simulink model "Simulatore_COMAU_UBHAND_IV_manipolazione_interna"  (Simulink 7.6)
settings: Solver=ode45  StartTime=0.0  StopTime=inf  RelTol=1e-3
values from neighbouring files (symbols this model uses):
  a_f = zeros(4,5)    [KinematicData.m]
  alpha_f = zeros(4,5)    [KinematicData.m]
  d_f = zeros(4,5)    [KinematicData.m]
  dc4_1 = 660    [KinematicData.m]
  T6HAND = [1 0 0 0;0 1 0 0; 0 0 1 357.48+95; 0 0 0 1]    [KinematicData.m]
  teta_d = atan(647.07/130)    [KinematicData.m]
  teta_f = zeros(4,5)    [KinematicData.m]
undefined symbols (not defined in the file or in its folder): a_c, a_w, alpha_c, alpha_w, d_c, d_w, Dt_fingers, teta_c
libraries: simulink x281, dspmtrx3 x171, aerolibutil x163, aerolibconvert2 x64, simulink_extras x46, vrlib x26, aerolibtransform2 x7, dspnetwork x1
(part 4 of 15)

== system Simulatore_COMAU_UBHAND_IV_manipolazione_interna/SCOPES/Direct_kinematic2/Direct_kinematic_hand/Direct_kinematic_thumb ==
  Joints_reference : Inport  PortDimensions=3
  T_0f : Inport  Port=2
  Add{,1..4} (5 alike) : Sum
  Constant : Constant  Value=[alpha_f(1,1) alpha_f(1,2) alpha_f(1,3) alpha_f(1,4)]
  Constant1 : Constant  Value=teta_f(1,1)
  Constant2 : Constant  Value=[a_f(1,1) a_f(1,2) a_f(1,3) a_f(1,4)]
  Constant3 : Constant  Value=teta_f(1,2)
  Constant4 : Constant  Value=teta_f(1,3)
  Constant5 : Constant  Value=teta_f(1,4)
  Constant6 : Constant  Value=-20
  Degrees to Radians : simulink_extras/Transformations/Degrees to Radians
  Demux : Demux
  Demux1 : Demux
  Demux2 : Demux
  Demux3 : Demux  Outputs=3
  Display3 : Display
  Gain : Gain  Gain=0.8
  Matrix Multiply{,1..3} (4 alike) : Product  Multiplication=Matrix(*)
  Subsystem : Subsystem
  Subsystem1 : Subsystem
  Subsystem2 : Subsystem
  Subsystem3 : Subsystem
  Vector Concatenate : Concatenate  Mode=Vector
  T_finger_tip : Outport
  T_thumb_base : Outport  Port=2
  wires:
  Constant/1 -> Demux1/1
  Subsystem/1(Out1) -> Matrix Multiply3/2
  Subsystem1/1(Out1) -> Matrix Multiply/2
  Matrix Multiply/1 -> Matrix Multiply1/1
  Subsystem2/1(Out1) -> Matrix Multiply1/2
  Subsystem3/1(Out1) -> Matrix Multiply2/2
  Matrix Multiply1/1 -> Matrix Multiply2/1
  Matrix Multiply2/1 -> T_finger_tip/1, T_thumb_base/1
  Constant2/1 -> Demux2/1
  Demux2/1 -> Subsystem/3(a)
  Demux2/2 -> Subsystem1/3(a)
  Demux2/3 -> Subsystem2/3(a)
  Demux2/4 -> Subsystem3/3(a)
  Demux1/1 -> Subsystem/2(alpha)
  Demux1/2 -> Subsystem1/2(alpha)
  Demux1/3 -> Subsystem2/2(alpha)
  Demux1/4 -> Subsystem3/2(alpha)
  Joints_reference/1 -> Demux3/1, Vector Concatenate/1
  Demux3/3 -> Gain/1
  Vector Concatenate/1 -> Degrees to Radians/1, Display3/1
  Degrees to Radians/1 -> Demux/1
  T_0f/1 -> Matrix Multiply3/1
  Matrix Multiply3/1 -> Matrix Multiply/1
  Constant1/1 -> Add/2
  Demux/1 -> Add/1
  Demux/2 -> Add1/1
  Demux/3 -> Add2/1
  Demux/4 -> Add3/1
  Add3/1 -> Subsystem3/1(q)
  Add2/1 -> Subsystem2/1(q)
  Add1/1 -> Subsystem1/1(q)
  Add/1 -> Subsystem/1(q)
  Constant3/1 -> Add1/2
  Constant4/1 -> Add2/2
  Constant5/1 -> Add3/2
  Constant6/1 -> Add4/2
  Gain/1 -> Add4/1
  Add4/1 -> Vector Concatenate/2

== system Simulatore_COMAU_UBHAND_IV_manipolazione_interna/SCOPES/Direct_kinematic2/Direct_kinematic_wrist/T_0_8 ==
  wrist_angles[1..2] : Inport
  T_0HAND : Inport  Port=2
  Add : Sum
  Add1 : Sum
  Constant : Constant  Value=[alpha_w(1) alpha_w(2)]
  Constant1 : Constant  Value=0
  Constant2 : Constant  Value=[a_w(1) a_w(2)]
  Constant3 : Constant  Value=0
  Degrees to Radians : simulink_extras/Transformations/Degrees to Radians
  Demux : Demux  Outputs=2
  Demux1 : Demux  Outputs=2
  Demux2 : Demux  Outputs=2
  Display3 : Display
  Matrix Multiply : Product  Multiplication=Matrix(*)
  Matrix Multiply1 : Product  Multiplication=Matrix(*)
  Matrix Multiply2 : Product  Multiplication=Matrix(*)
  Subsystem : Subsystem
  Subsystem1 : Subsystem
  wrist_reference_ : Subsystem
  T_08 : Outport
  wires:
  wrist_angles[1..2]/1 -> Display3/1, Degrees to Radians/1
  Degrees to Radians/1 -> Demux/1
  Demux1/2 -> Subsystem1/2(alpha)
  Demux1/1 -> Subsystem/2(alpha)
  Demux2/2 -> Subsystem1/3(a)
  Demux2/1 -> Subsystem/3(a)
  Constant2/1 -> Demux2/1
  Matrix Multiply/1 -> T_08/1
  Subsystem1/1(Out1) -> Matrix Multiply/2
  Constant/1 -> Demux1/1
  Subsystem/1(Out1) -> Matrix Multiply1/2
  Matrix Multiply1/1 -> Matrix Multiply/1
  Constant1/1 -> Add/2
  Demux/1 -> Add/1
  Add/1 -> Subsystem/1(q)
  Constant3/1 -> Add1/2
  Demux/2 -> Add1/1
  Add1/1 -> Subsystem1/1(q)
  T_0HAND/1 -> Matrix Multiply2/1
  wrist_reference_/1(T0_W) -> Matrix Multiply2/2
  Matrix Multiply2/1 -> Matrix Multiply1/1

== system Simulatore_COMAU_UBHAND_IV_manipolazione_interna/VISUALIZATION/Subsystem3/Subsystem1/Wrist ==
  xyz_0 : Inport
  Constant : Constant  Value=0
  Constant1 : Constant  Value=0
  Constant2 : Constant  Value=0
  Demux : Demux  Outputs=3
  Display : Display
  Display1 : Display
  Gain : Gain  Gain=-1
  Gain1 : Gain  Gain=-1
  Gain2 : Gain
  Mux : Mux  Inputs=3
  Sum : Sum
  Sum1 : Sum
  Sum2 : Sum
  xyz_vrl : Outport
  wires:
  Gain1/1 -> Mux/1
  Gain2/1 -> Mux/2
  Gain/1 -> Mux/3
  Sum2/1 -> Gain2/1
  Sum1/1 -> Gain1/1
  Sum/1 -> Gain/1
  Mux/1 -> Display/1, xyz_vrl/1
  Constant1/1 -> Sum1/1
  Demux/2 -> Sum1/2
  Constant2/1 -> Sum2/1
  Demux/3 -> Sum2/2
  Constant/1 -> Sum/1
  Demux/1 -> Sum/2
  xyz_0/1 -> Demux/1, Display1/1

== system Simulatore_COMAU_UBHAND_IV_manipolazione_interna/VISUALIZATION/Subsystem3/Subsystem2/Subsystem ==
  carpale_in : Inport
  prossimale_in : Inport  Port=2
  mediale_in : Inport  Port=3
  Angle Conversion : aerolibconvert2/Angle Conversion  IU=deg  OU=rad
  Angle Conversion1 : aerolibconvert2/Angle Conversion  IU=deg  OU=rad
  Constant : Constant
  Constant1 : Constant  Value=-1
  Constant{2..5} (4 alike) : Constant  Value=0
  Constant6 : Constant  Value=-90
  Constant7 : Constant  Value=20
  Gain : Gain  Gain=0.8
  Mux{1..4} (4 alike) : Mux
  Sum : Sum  Inputs=|++
  Sum1 : Sum  Inputs=|+-
  carpale_out : Outport
  prossimale_out : Outport  Port=2
  mediale_out : Outport  Port=3
  distale_out : Outport  Port=4
  wires:
  Constant/1 -> [uno]
  Constant1/1 -> [meno_uno]
  mediale_in/1 -> Mux3/4, Gain/1
  [uno] -> Mux3/2
  Constant4/1 -> Mux3/1, Mux3/3
  Mux3/1 -> mediale_out/1
  [uno] -> Mux4/2
  Constant5/1 -> Mux4/1, Mux4/3
  Mux4/1 -> distale_out/1
  [uno] -> Mux1/1
  Constant2/1 -> Mux1/3, Mux1/2
  Sum/1 -> Mux1/4
  Constant6/1 -> Angle Conversion/1
  prossimale_in/1 -> Sum/1
  Mux1/1 -> prossimale_out/1
  [meno_uno] -> Mux2/3
  carpale_in/1 -> Mux2/4
  Constant3/1 -> Mux2/1, Mux2/2
  Mux2/1 -> carpale_out/1
  Constant7/1 -> Angle Conversion1/1
  Gain/1 -> Sum1/1
  Sum1/1 -> Mux4/4
  Angle Conversion/1 -> Sum/2
  Angle Conversion1/1 -> Sum1/2

== system Simulatore_COMAU_UBHAND_IV_manipolazione_interna/VISUALIZATION/Subsystem3/Subsystem2/Subsystem1 ==
  carpale_in : Inport
  prossimale_in : Inport  Port=2
  mediale_in : Inport  Port=3
  Constant : Constant
  Constant1 : Constant  Value=-1
  Constant{2..5} (4 alike) : Constant  Value=0
  Display : Display
  Gain : Gain  Gain=0.7
  Mux{1..4} (4 alike) : Mux
  carpale_out : Outport
  prossimale_out : Outport  Port=2
  mediale_out : Outport  Port=3
  distale_out : Outport  Port=4
  wires:
  Mux1/1 -> carpale_out/1
  Mux2/1 -> prossimale_out/1
  Constant/1 -> [uno]
  Constant1/1 -> [meno_uno]
  Constant2/1 -> Mux1/1, Mux1/3
  carpale_in/1 -> Mux1/4
  prossimale_in/1 -> Mux2/4
  mediale_in/1 -> Mux3/4, Gain/1
  Constant4/1 -> Mux3/2, Mux3/3
  Mux3/1 -> mediale_out/1
  Constant5/1 -> Mux4/3, Mux4/2
  Gain/1 -> Mux4/4
  Mux4/1 -> distale_out/1, Display/1
  [meno_uno] -> Mux1/2
  [uno] -> Mux2/1
  Constant3/1 -> Mux2/2, Mux2/3
  [uno] -> Mux3/1
  [uno] -> Mux4/1

== system Simulatore_COMAU_UBHAND_IV_manipolazione_interna/VISUALIZATION/Subsystem3/Subsystem2/Subsystem2 ==
  carpale_in : Inport
  prossimale_in : Inport  Port=2
  mediale_in : Inport  Port=3
  Constant : Constant
  Constant1 : Constant  Value=-1
  Constant{2..5} (4 alike) : Constant  Value=0
  Gain : Gain  Gain=0.7
  Mux{1..4} (4 alike) : Mux
  carpale_out : Outport
  prossimale_out : Outport  Port=2
  mediale_out : Outport  Port=3
  distale_out : Outport  Port=4
  wires:
  Constant/1 -> [uno]
  Constant1/1 -> [meno_uno]
  Mux1/1 -> carpale_out/1
  Mux2/1 -> prossimale_out/1
  Constant2/1 -> Mux1/1, Mux1/3
  carpale_in/1 -> Mux1/4
  prossimale_in/1 -> Mux2/4
  mediale_in/1 -> Mux3/4, Gain/1
  Constant4/1 -> Mux3/2, Mux3/3
  Mux3/1 -> mediale_out/1
  Constant5/1 -> Mux4/3, Mux4/2
  Gain/1 -> Mux4/4
  Mux4/1 -> distale_out/1
  [meno_uno] -> Mux1/2
  [uno] -> Mux2/1
  Constant3/1 -> Mux2/2, Mux2/3
  [uno] -> Mux3/1
  [uno] -> Mux4/1

== system Simulatore_COMAU_UBHAND_IV_manipolazione_interna/VISUALIZATION/Subsystem3/Subsystem2/Subsystem3 ==
  carpale_in : Inport
  prossimale_in : Inport  Port=2
  mediale_in : Inport  Port=3
  Constant : Constant
  Constant1 : Constant  Value=-1
  Constant{2..5} (4 alike) : Constant  Value=0
  Gain : Gain  Gain=0.7
  Mux{1..4} (4 alike) : Mux
  carpale_out : Outport
  prossimale_out : Outport  Port=2
  mediale_out : Outport  Port=3
  distale_out : Outport  Port=4
  wires:
  Constant/1 -> [uno]
  Constant1/1 -> [meno_uno]
  Mux1/1 -> carpale_out/1
  Mux2/1 -> prossimale_out/1
  Constant2/1 -> Mux1/1, Mux1/3
  carpale_in/1 -> Mux1/4
  prossimale_in/1 -> Mux2/4
  mediale_in/1 -> Mux3/4, Gain/1
  Constant4/1 -> Mux3/2, Mux3/3
  Mux3/1 -> mediale_out/1
  Constant5/1 -> Mux4/3, Mux4/2
  Gain/1 -> Mux4/4
  Mux4/1 -> distale_out/1
  [meno_uno] -> Mux1/2
  [uno] -> Mux2/1
  Constant3/1 -> Mux2/2, Mux2/3
  [uno] -> Mux3/1
  [uno] -> Mux4/1

== system Simulatore_COMAU_UBHAND_IV_manipolazione_interna/VISUALIZATION/Subsystem3/Subsystem2/Subsystem4 ==
  carpale_in : Inport
  prossimale_in : Inport  Port=2
  mediale_in : Inport  Port=3
  Constant : Constant
  Constant1 : Constant  Value=-1
  Constant{2..5} (4 alike) : Constant  Value=0
  Gain : Gain  Gain=0.7
  Mux{1..4} (4 alike) : Mux
  carpale_out : Outport
  prossimale_out : Outport  Port=2
  mediale_out : Outport  Port=3
  distale_out : Outport  Port=4
  wires:
  Constant/1 -> [uno]
  Constant1/1 -> [meno_uno]
  Mux1/1 -> carpale_out/1
  Mux2/1 -> prossimale_out/1
  Constant2/1 -> Mux1/1, Mux1/3
  carpale_in/1 -> Mux1/4
  prossimale_in/1 -> Mux2/4
  mediale_in/1 -> Mux3/4, Gain/1
  Constant4/1 -> Mux3/2, Mux3/3
  Mux3/1 -> mediale_out/1
  Constant5/1 -> Mux4/3, Mux4/2
  Gain/1 -> Mux4/4
  Mux4/1 -> distale_out/1
  [meno_uno] -> Mux1/2
  [uno] -> Mux2/1
  Constant3/1 -> Mux2/2, Mux2/3
  [uno] -> Mux3/1
  [uno] -> Mux4/1

== system Simulatore_COMAU_UBHAND_IV_manipolazione_interna/VISUALIZATION/Subsystem3/Subsystem2/Subsystem5 ==
  wrist 1_in : Inport
  wrist 2_in : Inport  Port=2
  Constant : Constant
  Constant1 : Constant  Value=-1
  Constant2 : Constant  Value=0
  Constant3 : Constant  Value=0
  Mux1 : Mux
  Mux2 : Mux
  wrist 1_out : Outport
  wrist 2_out : Outport  Port=2
  wires:
  Constant3/1 -> Mux2/3, Mux2/2
  wrist 2_in/1 -> Mux2/4
  wrist 1_in/1 -> Mux1/4
  Constant2/1 -> Mux1/3, Mux1/2
  Constant1/1 -> [meno_uno]
  Constant/1 -> [uno]
  Mux2/1 -> wrist 2_out/1
  Mux1/1 -> wrist 1_out/1
  [uno] -> Mux1/1
  [meno_uno] -> Mux2/1

== system Simulatore_COMAU_UBHAND_IV_manipolazione_interna/VISUALIZATION/Subsystem3/finger_tip_xyz/world_frame2vrl_frame ==
  xyz_0 : Inport
  Constant : Constant  Value=0
  Constant1 : Constant  Value=0
  Constant2 : Constant  Value=0
  Demux : Demux  Outputs=3
  Display : Display
  Display1 : Display
  Gain : Gain  Gain=-1
  Gain1 : Gain  Gain=-1
  Gain2 : Gain
  Mux : Mux  Inputs=3
  Sum : Sum
  Sum1 : Sum
  Sum2 : Sum
  xyz_vrl : Outport
  wires:
  Gain1/1 -> Mux/1
  Gain2/1 -> Mux/2
  Gain/1 -> Mux/3
  Sum2/1 -> Gain2/1
  Sum1/1 -> Gain1/1
  Sum/1 -> Gain/1
  Mux/1 -> Display/1, xyz_vrl/1
  Constant1/1 -> Sum1/1
  Demux/2 -> Sum1/2
  Constant2/1 -> Sum2/1
  Demux/3 -> Sum2/2
  Constant/1 -> Sum/1
  Demux/1 -> Sum/2
  xyz_0/1 -> Demux/1, Display1/1

== system Simulatore_COMAU_UBHAND_IV_manipolazione_interna/VISUALIZATION/Subsystem3/finger_tip_xyz/world_frame2vrl_frame1 ==
  xyz_0 : Inport
  Constant : Constant  Value=0
  Constant1 : Constant  Value=0
  Constant2 : Constant  Value=0
  Demux : Demux  Outputs=3
  Display : Display
  Display1 : Display
  Gain : Gain  Gain=-1
  Gain1 : Gain  Gain=-1
  Gain2 : Gain
  Mux : Mux  Inputs=3
  Sum : Sum
  Sum1 : Sum
  Sum2 : Sum
  xyz_vrl : Outport
  wires:
  xyz_0/1 -> Display1/1, Demux/1
  Demux/1 -> Sum/2
  Constant/1 -> Sum/1
  Demux/3 -> Sum2/2
  Constant2/1 -> Sum2/1
  Demux/2 -> Sum1/2
  Constant1/1 -> Sum1/1
  Mux/1 -> xyz_vrl/1, Display/1
  Sum/1 -> Gain/1
  Sum1/1 -> Gain1/1
  Sum2/1 -> Gain2/1
  Gain/1 -> Mux/3
  Gain2/1 -> Mux/2
  Gain1/1 -> Mux/1

== system Simulatore_COMAU_UBHAND_IV_manipolazione_interna/VISUALIZATION/Subsystem3/finger_tip_xyz/world_frame2vrl_frame2 ==
  xyz_0 : Inport
  Constant : Constant  Value=0
  Constant1 : Constant  Value=0
  Constant2 : Constant  Value=0
  Demux : Demux  Outputs=3
  Display : Display
  Display1 : Display
  Gain : Gain  Gain=-1
  Gain1 : Gain  Gain=-1
  Gain2 : Gain
  Mux : Mux  Inputs=3
  Sum : Sum
  Sum1 : Sum
  Sum2 : Sum
  xyz_vrl : Outport
  wires:
  Gain1/1 -> Mux/1
  Gain2/1 -> Mux/2
  Gain/1 -> Mux/3
  Sum2/1 -> Gain2/1
  Sum1/1 -> Gain1/1
  Sum/1 -> Gain/1
  Mux/1 -> Display/1, xyz_vrl/1
  Constant1/1 -> Sum1/1
  Demux/2 -> Sum1/2
  Constant2/1 -> Sum2/1
  Demux/3 -> Sum2/2
  Constant/1 -> Sum/1
  Demux/1 -> Sum/2
  xyz_0/1 -> Demux/1, Display1/1

== system Simulatore_COMAU_UBHAND_IV_manipolazione_interna/VISUALIZATION/Subsystem3/finger_tip_xyz/world_frame2vrl_frame3 ==
  xyz_0 : Inport
  Constant : Constant  Value=0
  Constant1 : Constant  Value=0
  Constant2 : Constant  Value=0
  Demux : Demux  Outputs=3
  Display : Display
  Display1 : Display
  Gain : Gain  Gain=-1
  Gain1 : Gain  Gain=-1
  Gain2 : Gain
  Mux : Mux  Inputs=3
  Sum : Sum
  Sum1 : Sum
  Sum2 : Sum
  xyz_vrl : Outport
  wires:
  xyz_0/1 -> Display1/1, Demux/1
  Demux/1 -> Sum/2
  Constant/1 -> Sum/1
  Demux/3 -> Sum2/2
  Constant2/1 -> Sum2/1
  Demux/2 -> Sum1/2
  Constant1/1 -> Sum1/1
  Mux/1 -> xyz_vrl/1, Display/1
  Sum/1 -> Gain/1
  Sum1/1 -> Gain1/1
  Sum2/1 -> Gain2/1
  Gain/1 -> Mux/3
  Gain2/1 -> Mux/2
  Gain1/1 -> Mux/1

== system Simulatore_COMAU_UBHAND_IV_manipolazione_interna/VISUALIZATION/Subsystem3/finger_tip_xyz/world_frame2vrl_frame4 ==
  xyz_0 : Inport
  Constant : Constant  Value=0
  Constant1 : Constant  Value=0
  Constant2 : Constant  Value=0
  Demux : Demux  Outputs=3
  Display : Display
  Display1 : Display
  Gain : Gain  Gain=-1
  Gain1 : Gain  Gain=-1
  Gain2 : Gain
  Mux : Mux  Inputs=3
  Sum : Sum
  Sum1 : Sum
  Sum2 : Sum
  xyz_vrl : Outport
  wires:
  Gain1/1 -> Mux/1
  Gain2/1 -> Mux/2
  Gain/1 -> Mux/3
  Sum2/1 -> Gain2/1
  Sum1/1 -> Gain1/1
  Sum/1 -> Gain/1
  Mux/1 -> Display/1, xyz_vrl/1
  Constant1/1 -> Sum1/1
  Demux/2 -> Sum1/2
  Constant2/1 -> Sum2/1
  Demux/3 -> Sum2/2
  Constant/1 -> Sum/1
  Demux/1 -> Sum/2
  xyz_0/1 -> Demux/1, Display1/1

== system Simulatore_COMAU_UBHAND_IV_manipolazione_interna/Work Space Control/Grasp Planner/SCOPES/Direct_kinematic2 ==
  q[1..6] : Inport
  wrist_angles[1..2] : Inport  Port=2
  fingers_joints_references : Inport  Port=3
  Constant2 : Constant  Value=T6HAND  VectorParams1D=off
  Direct_kinematic_Comau : Subsystem
  Direct_kinematic_hand : Subsystem
  Direct_kinematic_wrist : Subsystem
  Matrix Multiply1 : Product  Multiplication=Matrix(*)
  Mux : Mux  Inputs=5
  T0HAND : Outport
  fingers_tips_xyz : Outport  Port=2
  wires:
  q[1..6]/1 -> Direct_kinematic_Comau/1(q[1..6])
  Direct_kinematic_Comau/1(T_0_6) -> Matrix Multiply1/1
  Constant2/1 -> Matrix Multiply1/2
  Matrix Multiply1/1 -> Direct_kinematic_wrist/2(T_0HAND)
  wrist_angles[1..2]/1 -> Direct_kinematic_wrist/1(wrist_angles[1..2])
  fingers_joints_references/1 -> Direct_kinematic_hand/1(Joint_references)
  Direct_kinematic_wrist/1(T_08) -> Direct_kinematic_hand/2(T_08), T0HAND/1
  Direct_kinematic_hand/1(T_finger_tip_thumb) -> Mux/1
  Direct_kinematic_hand/2(T_finger_tip_index) -> Mux/2
  Direct_kinematic_hand/3(T_finger_tip_middle) -> Mux/3
  Direct_kinematic_hand/4(T_finger_tip_ring) -> Mux/4
  Direct_kinematic_hand/5(T_finger_tip_little) -> Mux/5
  Mux/1 -> fingers_tips_xyz/1

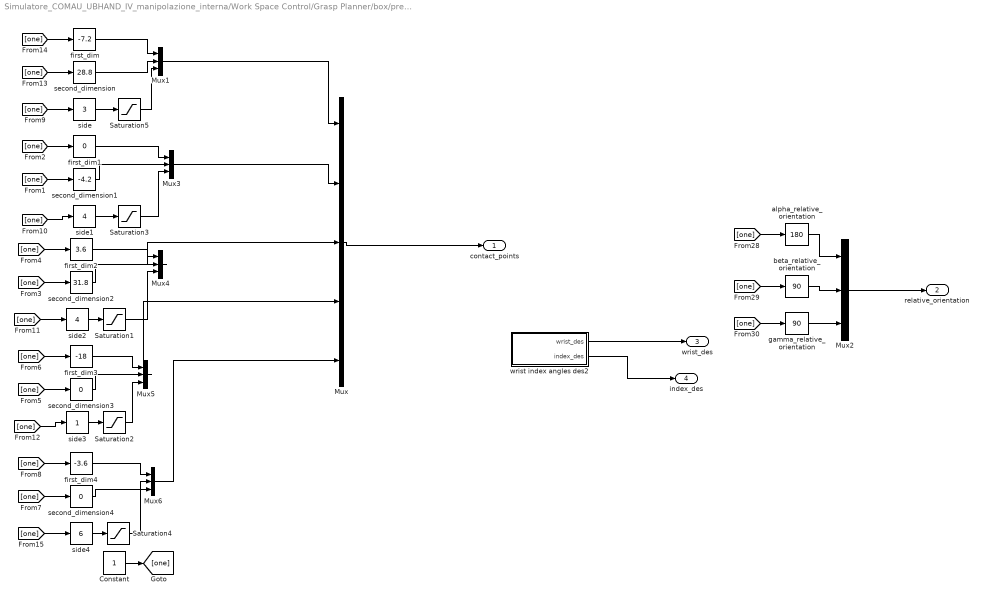
== system Simulatore_COMAU_UBHAND_IV_manipolazione_interna/Work Space Control/Grasp Planner/box/presa da destra ==
  Constant : Constant
  Mux : Mux  Inputs=5
  Mux{1..6} (6 alike) : Mux  Inputs=3
  Saturation{1..5} (5 alike) : Saturate  UpperLimit=6  LowerLimit=1
  alpha_relative_orientation : simulink/Math Operations/Slider Gain  low=-180  gain=180  high=180
  beta_relative_orientation : simulink/Math Operations/Slider Gain  low=-180  gain=90  high=180
  first_dim : simulink/Math Operations/Slider Gain  low=-180  gain=-7.2  high=180
  first_dim1 : simulink/Math Operations/Slider Gain  low=-180  gain=0  high=180
  first_dim2 : simulink/Math Operations/Slider Gain  low=-180  gain=3.6  high=180
  first_dim3 : simulink/Math Operations/Slider Gain  low=-180  gain=-18  high=180
  first_dim4 : simulink/Math Operations/Slider Gain  low=-180  gain=-3.6  high=180
  gamma_relative_orientation : simulink/Math Operations/Slider Gain  low=-180  gain=90  high=180
  second_dimension : simulink/Math Operations/Slider Gain  low=-180  gain=28.8  high=180
  second_dimension1 : simulink/Math Operations/Slider Gain  low=-180  gain=-4.2  high=180
  second_dimension2 : simulink/Math Operations/Slider Gain  low=-180  gain=31.8  high=180
  second_dimension3 : simulink/Math Operations/Slider Gain  low=-180  gain=0  high=180
  second_dimension4 : simulink/Math Operations/Slider Gain  low=-180  gain=0  high=180
  side : simulink/Math Operations/Slider Gain  low=-94  gain=3  high=6
  side1 : simulink/Math Operations/Slider Gain  low=-94  gain=4  high=6
  side2 : simulink/Math Operations/Slider Gain  low=-94  gain=4  high=6
  side3 : simulink/Math Operations/Slider Gain  low=-94  gain=1  high=6
  side4 : simulink/Math Operations/Slider Gain  low=-94  gain=6  high=6
  wrist index angles des2 : Subsystem
  contact_points : Outport
  relative_orientation : Outport  Port=2
  wrist_des : Outport  Port=3
  index_des : Outport  Port=4
  wires:
  Mux2/1 -> relative_orientation/1
  gamma_relative_orientation/1 -> Mux2/3
  beta_relative_orientation/1 -> Mux2/2
  alpha_relative_orientation/1 -> Mux2/1
  [one] -> gamma_relative_orientation/1
  [one] -> beta_relative_orientation/1
  [one] -> alpha_relative_orientation/1
  Constant/1 -> [one]
  wrist index angles des2/1(wrist_des) -> wrist_des/1
  wrist index angles des2/2(index_des) -> index_des/1
  Mux/1 -> contact_points/1
  [one] -> second_dimension/1
  [one] -> first_dim/1
  first_dim/1 -> Mux1/1
  second_dimension/1 -> Mux1/2
  Mux1/1 -> Mux/1
  side/1 -> Saturation5/1
  [one] -> second_dimension1/1
  [one] -> first_dim1/1
  first_dim1/1 -> Mux3/1
  second_dimension1/1 -> Mux3/2
  side1/1 -> Saturation3/1
  [one] -> second_dimension2/1
  [one] -> first_dim2/1
  first_dim2/1 -> Mux4/1
  second_dimension2/1 -> Mux4/2
  side2/1 -> Saturation1/1
  [one] -> second_dimension3/1
  [one] -> first_dim3/1
  first_dim3/1 -> Mux5/1
  second_dimension3/1 -> Mux5/2
  side3/1 -> Saturation2/1
  [one] -> second_dimension4/1
  [one] -> first_dim4/1
  first_dim4/1 -> Mux6/1
  second_dimension4/1 -> Mux6/2
  side4/1 -> Saturation4/1
  Mux3/1 -> Mux/2
  Mux4/1 -> Mux/3
  Mux5/1 -> Mux/4
  Mux6/1 -> Mux/5
  [one] -> side/1
  [one] -> side1/1
  [one] -> side2/1
  [one] -> side3/1
  [one] -> side4/1
  Saturation4/1 -> Mux6/3
  Saturation2/1 -> Mux5/3
  Saturation1/1 -> Mux4/3
  Saturation3/1 -> Mux3/3
  Saturation5/1 -> Mux1/3

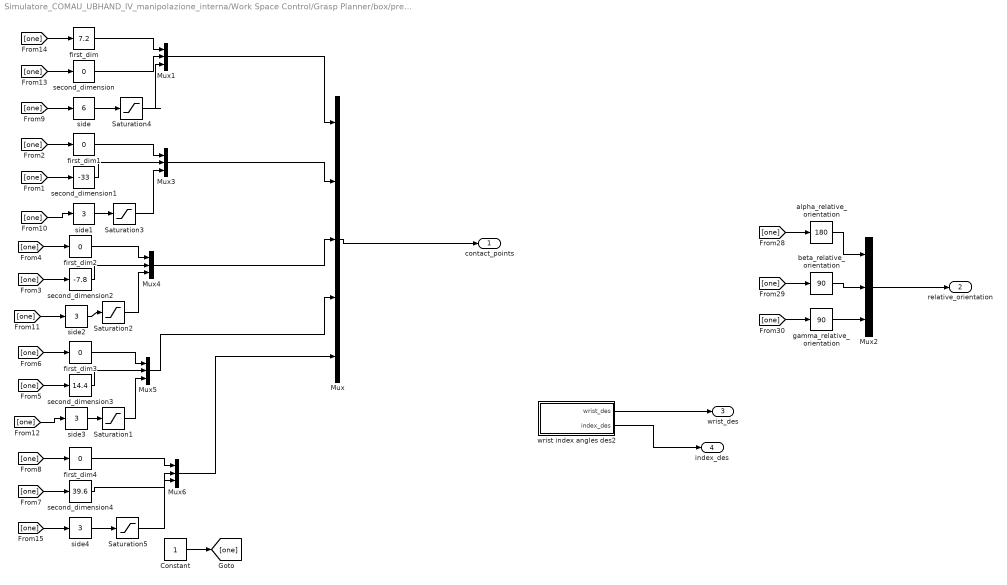
== system Simulatore_COMAU_UBHAND_IV_manipolazione_interna/Work Space Control/Grasp Planner/box/presa dal basso ==
  Constant : Constant
  Mux : Mux  Inputs=5
  Mux{1..6} (6 alike) : Mux  Inputs=3
  Saturation{1..5} (5 alike) : Saturate  UpperLimit=1  LowerLimit=0
  alpha_relative_orientation : simulink/Math Operations/Slider Gain  low=-180  gain=180  high=180
  beta_relative_orientation : simulink/Math Operations/Slider Gain  low=-180  gain=90  high=180
  first_dim : simulink/Math Operations/Slider Gain  low=-180  gain=7.2  high=180
  first_dim{1..4} (4 alike) : simulink/Math Operations/Slider Gain  low=-180  gain=0  high=180
  gamma_relative_orientation : simulink/Math Operations/Slider Gain  low=-180  gain=90  high=180
  second_dimension : simulink/Math Operations/Slider Gain  low=-180  gain=0  high=180
  second_dimension1 : simulink/Math Operations/Slider Gain  low=-180  gain=-33  high=180
  second_dimension2 : simulink/Math Operations/Slider Gain  low=-180  gain=-7.8  high=180
  second_dimension3 : simulink/Math Operations/Slider Gain  low=-180  gain=14.4  high=180
  second_dimension4 : simulink/Math Operations/Slider Gain  low=-180  gain=39.6  high=180
  side : simulink/Math Operations/Slider Gain  low=-94  gain=6  high=6
  side{1..4} (4 alike) : simulink/Math Operations/Slider Gain  low=-94  gain=3  high=6
  wrist index angles des2 : Subsystem
  contact_points : Outport
  relative_orientation : Outport  Port=2
  wrist_des : Outport  Port=3
  index_des : Outport  Port=4
  wires:
  [one] -> alpha_relative_orientation/1
  [one] -> beta_relative_orientation/1
  [one] -> gamma_relative_orientation/1
  alpha_relative_orientation/1 -> Mux2/1
  beta_relative_orientation/1 -> Mux2/2
  gamma_relative_orientation/1 -> Mux2/3
  Mux2/1 -> relative_orientation/1
  Constant/1 -> [one]
  wrist index angles des2/1(wrist_des) -> wrist_des/1
  wrist index angles des2/2(index_des) -> index_des/1
  Mux/1 -> contact_points/1
  [one] -> second_dimension/1
  [one] -> first_dim/1
  first_dim/1 -> Mux1/1
  second_dimension/1 -> Mux1/2
  Mux1/1 -> Mux/1
  side/1 -> Saturation4/1
  [one] -> second_dimension1/1
  [one] -> first_dim1/1
  first_dim1/1 -> Mux3/1
  second_dimension1/1 -> Mux3/2
  side1/1 -> Saturation3/1
  [one] -> second_dimension2/1
  [one] -> first_dim2/1
  first_dim2/1 -> Mux4/1
  second_dimension2/1 -> Mux4/2
  side2/1 -> Saturation2/1
  [one] -> second_dimension3/1
  [one] -> first_dim3/1
  first_dim3/1 -> Mux5/1
  second_dimension3/1 -> Mux5/2
  side3/1 -> Saturation1/1
  [one] -> second_dimension4/1
  [one] -> first_dim4/1
  first_dim4/1 -> Mux6/1
  second_dimension4/1 -> Mux6/2
  side4/1 -> Saturation5/1
  Mux3/1 -> Mux/2
  Mux4/1 -> Mux/3
  Mux5/1 -> Mux/4
  Mux6/1 -> Mux/5
  [one] -> side/1
  [one] -> side1/1
  [one] -> side2/1
  [one] -> side3/1
  [one] -> side4/1
  Saturation4/1 -> Mux1/3
  Saturation3/1 -> Mux3/3
  Saturation2/1 -> Mux4/3
  Saturation1/1 -> Mux5/3
  Saturation5/1 -> Mux6/3
